simulink model "Demo_PreScanCarRealTime2D_cs"  (Simulink 8.0)
settings: Solver=ode5  SolverMode=SingleTasking  StartTime=0.0  StopTime=inf  FixedStep=1/1000  RelTol=1e-3
PreLoadFcn:
  | check_NUTS_defined;
  | assignin('base',['model_closed_' bdroot],false);
  | addpath('../includes_AEBS');
InitFcn:
  | PreScanExperimentInitFcn(bdroot)
StopFcn:
  | PreScanExperimentStopFcn(bdroot);
  | 
undefined symbols (not defined in the file or in its folder): DiscIntNeverNeededParam, Driver_Demands, vicrt_initcallback, vicrt_initmexcallback, vicrt_simulationmanager, x_ini_VRML, y_ini_VRML, z_ini_VRML
libraries: PreScanSimulinkLibrary x48, PreScanUsefulBlocks x15, simulink x15, simulink_extras x12, simulink_need_slupdate x1, dspsigops x1
(part 14 of 16)

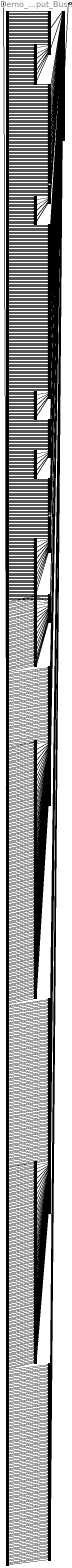
== system Demo_PreScanCarRealTime2D_cs/HostVehicle/Dynamics_UserSpecified/VI-CarRealTime/Output_Bus/IOmap2bus ==
  In : Inport
  Actual_Tread_Speed : BusCreator
  Aggregate_Force_At_Wheel : BusCreator
  Alpha : BusCreator
  Animator_Widget : BusCreator  Inputs=2
  Auxiliary_Roll_Force : BusCreator
  Auxiliary_Roll_Force_at_element : BusCreator  Inputs=2
  Auxiliary_Roll_deformation_at_element : BusCreator  Inputs=2
  Brake : BusCreator  Inputs=7
  Brake_Moment : BusCreator
  Caster_Angle : BusCreator  Inputs=2
  Chamber_Pressure : BusCreator
  Contact_Patch_Global_X : BusCreator
  Contact_Patch_Global_Y : BusCreator
  Contact_Patch_Global_Z : BusCreator
  Contact_Patch_Global_dZdX : BusCreator
  Contact_Patch_Global_dZdY : BusCreator
  Damper : BusCreator  Inputs=5
  Demux : Demux  Outputs=1108
  Drive_Moment : BusCreator
  Effective_Closing_Speed : BusCreator
  Effective_Penetration : BusCreator
  Effective_Rolling_Radius : BusCreator
  Equivalent_Tread_Speed : BusCreator
  Euler_Roll : BusCreator
  External_Friction_Scale_Factor : BusCreator
  Force_At_Bumper : BusCreator
  Force_At_Bumper1 : BusCreator
  Force_At_Damper : BusCreator
  Force_At_Spring : BusCreator
  Force_At_Wheel{,1..3} (4 alike) : BusCreator
  Force_Aux_At_Wheel : BusCreator
  Global_Omega_X : BusCreator
  Global_Omega_Y : BusCreator
  Global_Omega_Z : BusCreator
  Global_Vx : BusCreator
  Global_Vy : BusCreator
  Global_Vz : BusCreator
  Global_X : BusCreator
  Global_Y : BusCreator
  Global_Z : BusCreator
  Ground_Surface_Contact_Patch_Vx : BusCreator
  Ground_Surface_Contact_Patch_Vy : BusCreator
  Ground_Surface_Force_X : BusCreator
  Ground_Surface_Force_Y : BusCreator
  Ground_Surface_Force_Z : BusCreator
  Ground_Surface_Gyroscopic_Moment : BusCreator
  Ground_Surface_Kappa_Fy : BusCreator
  Ground_Surface_Moment_X : BusCreator
  Ground_Surface_Moment_Y : BusCreator
  Ground_Surface_Moment_Z : BusCreator
  Ground_Surface_Peak_Friction_X : BusCreator
  Ground_Surface_Peak_Friction_Y : BusCreator
  Ground_Surface_Residual_Torque : BusCreator
  Ground_Surface_Wheel_Center_Vx : BusCreator
  Gyro : BusCreator  Inputs=10
  Hook_rear : BusCreator  Inputs=18
  Hub_Carrier_Force_Global_X : BusCreator
  Hub_Carrier_Force_Global_Y : BusCreator
  Hub_Carrier_Force_Global_Z : BusCreator
  Hub_Carrier_Force_Local_X : BusCreator
  Hub_Carrier_Force_Local_Y : BusCreator
  Hub_Carrier_Force_Local_Z : BusCreator
  Hub_Carrier_Moment_Global_X : BusCreator
  Hub_Carrier_Moment_Global_Y : BusCreator
  Hub_Carrier_Moment_Global_Z : BusCreator
  Hub_Carrier_Moment_Local_X : BusCreator
  Hub_Carrier_Moment_Local_Y : BusCreator
  Hub_Carrier_Moment_Local_Z : BusCreator
  Hub_Carrier_Omega_X : BusCreator
  Hub_Carrier_Omega_Y : BusCreator
  Hub_Carrier_Omega_Z : BusCreator
  Hub_Carrier_Vx : BusCreator
  Hub_Carrier_Vy : BusCreator
  Hub_Carrier_Vz : BusCreator
  Inclination : BusCreator
  Inst_Stiff_Deflection : BusCreator
  Inst_Stiff_Force : BusCreator
  Jounce{,1..4} (5 alike) : BusCreator
  Jounce_Acceleration{,1..4} (5 alike) : BusCreator
  Jounce_Bumper : BusCreator  Inputs=5
  Jounce_Rate{,1..4} (5 alike) : BusCreator
  Kingpin_Moment : BusCreator  Inputs=1
  Kingpin_Moment1 : BusCreator  Inputs=2
  Lateral_Relaxation_Length : BusCreator
  Lateral_Slip_With_Lag : BusCreator
  Lateral_Slip_Without_Lag : BusCreator
  Loaded_Radius : BusCreator
  Locked_Damper_Moment : BusCreator
  Locked_Moment : BusCreator
  Locked_Spring_Moment : BusCreator
  Locked_Switch : BusCreator
  Lockup_Rotation : BusCreator
  Longitudinal_Relaxation_Length : BusCreator
  Longitudinal_Slip_With_Lag : BusCreator
  Longitudinal_Slip_Without_Lag : BusCreator
  Moment_Arm_Of_Fx : BusCreator
  Omega : BusCreator
  OutputBus : BusCreator  Inputs=92
  PID_CW_At_Spring : BusCreator
  PID_PH_At_Spring : BusCreator
  PID_RH_At_Spring : BusCreator
  Phi : BusCreator
  Pitch : BusCreator
  Pneumatic_Trail : BusCreator
  Psi : BusCreator
  Rebound_Bumper : BusCreator  Inputs=5
  Roll : BusCreator  Inputs=2
  Rotation : BusCreator
  Sensor : BusCreator  Inputs=16
  Side_View_Angle : BusCreator
  Spindle_Steer : BusCreator
  Spring : BusCreator  Inputs=10
  Steering_Instant_Axis_Rotation : BusCreator  Inputs=2
  Steering_System : BusCreator  Inputs=6
  Suspension : BusCreator  Inputs=14
  System : BusCreator
  System_Mass_Center : BusCreator  Inputs=13
  Theta : BusCreator
  TieRod_Force_Left : BusCreator  Inputs=3
  TieRod_Force_Right : BusCreator  Inputs=3
  Tire : BusCreator  Inputs=46
  User_Input_Contact_Patch_Global_Z_Perturbation : BusCreator
  Vehicle : BusCreator  Inputs=46
  Vehicle_States : BusCreator  Inputs=20
  Wheel : BusCreator  Inputs=37
  Yaw : BusCreator
  aero_forces : BusCreator  Inputs=24
  base_acceleration : BusCreator
  base_deformation : BusCreator
  base_velocity : BusCreator
  bul_jounce_stop_data : BusCreator  Inputs=6
  bul_rebound_stop_data : BusCreator  Inputs=6
  bur_jounce_stop_data : BusCreator  Inputs=6
  bur_rebound_stop_data : BusCreator  Inputs=6
  bus_dmp_jounce_stop_data : BusCreator  Inputs=6
  bus_ins_jounce_stop_data : BusCreator  Inputs=6
  bus_roll_dmp_jounce_stop_data : BusCreator  Inputs=6
  bus_spr_jounce_stop_data : BusCreator  Inputs=6
  chassis_accelerations : BusCreator  Inputs=6
  chassis_displacements : BusCreator  Inputs=9
  chassis_velocities : BusCreator  Inputs=6
  clutch : BusCreator  Inputs=2
  condition_sensors : BusCreator  Inputs=1
  cp_force_lateralFL : BusCreator  Inputs=3
  cp_force_lateralFR : BusCreator  Inputs=3
  cp_force_lateralRL : BusCreator  Inputs=3
  cp_force_lateralRR : BusCreator  Inputs=3
  cp_force_longitudinalFL : BusCreator  Inputs=3
  cp_force_longitudinalFR : BusCreator  Inputs=3
  cp_force_longitudinalRL : BusCreator  Inputs=3
  cp_force_longitudinalRR : BusCreator  Inputs=3
  cp_force_normalFL : BusCreator  Inputs=3
  cp_force_normalFR : BusCreator  Inputs=3
  cp_force_normalRL : BusCreator  Inputs=3
  cp_force_normalRR : BusCreator  Inputs=3
  cross_weight : BusCreator
  dal_ride_damper_data : BusCreator
  dar_ride_damper_data : BusCreator
  das_ride_damper_data : BusCreator  Inputs=6
  das_roll_damper_data : BusCreator  Inputs=8
  das_third_damper_data : BusCreator  Inputs=6
  differential_speeds : BusCreator  Inputs=6
  differential_torques : BusCreator  Inputs=6
  driver_demands : BusCreator  Inputs=7
  driving_machine_monitor : BusCreator  Inputs=9
  engine : BusCreator  Inputs=7
  fuel_consumption : BusCreator
  ges_chassis_XFORM : BusCreator  Inputs=6
  ges_hub_XFORMFL : BusCreator  Inputs=6
  ges_hub_XFORMFR : BusCreator  Inputs=6
  ges_hub_XFORMRL : BusCreator  Inputs=6
  ges_hub_XFORMRR : BusCreator  Inputs=6
  hub2cp_XFORMFL : BusCreator  Inputs=3
  hub2cp_XFORMFR : BusCreator  Inputs=3
  hub2cp_XFORMRL : BusCreator  Inputs=3
  hub2cp_XFORMRR : BusCreator  Inputs=3
  hub_center_accx : BusCreator
  hub_center_accy : BusCreator
  hub_center_accz : BusCreator
  ins_inerter_data : BusCreator  Inputs=10
  jfs_torsional_stiffness_data : BusCreator  Inputs=12
  nsl_ride_spring_data : BusCreator  Inputs=6
  nsr_ride_spring_data : BusCreator  Inputs=6
  nss_ride_spring_data : BusCreator  Inputs=10
  res_dmp_rebound_stop_data : BusCreator  Inputs=6
  res_ins_rebound_stop_data : BusCreator  Inputs=6
  res_roll_dmp_rebound_stop_data : BusCreator  Inputs=6
  res_spr_rebound_stop_data : BusCreator  Inputs=6
  rhl_front_setup_sensor_data : BusCreator  Inputs=1
  rhr_front_setup_sensor_data : BusCreator  Inputs=1
  rhs_rear_setup_sensor_data : BusCreator  Inputs=1
  til_wheel_tire_forces : BusCreator  Inputs=18
  til_wheel_tire_kinematics : BusCreator  Inputs=9
  til_wheel_tire_rolling_states : BusCreator  Inputs=9
  tir_wheel_tire_forces : BusCreator  Inputs=18
  tir_wheel_tire_kinematics : BusCreator  Inputs=9
  tir_wheel_tire_rolling_states : BusCreator  Inputs=9
  transmission : BusCreator
  ues_third_spring_data : BusCreator  Inputs=6
  upright_accelerometer : BusCreator
  wheel_angles : BusCreator  Inputs=12
  wheel_center_dispx : BusCreator
  wheel_center_dispy : BusCreator
  wheel_center_dispz : BusCreator
  whl_front_wheel_XFORM : BusCreator  Inputs=9
  whl_rear_wheel_XFORM : BusCreator  Inputs=9
  whl_twin_rear_wheel_XFORM : BusCreator  Inputs=9
  whr_front_wheel_XFORM : BusCreator  Inputs=9
  whr_rear_wheel_XFORM : BusCreator  Inputs=9
  whr_twin_rear_wheel_XFORM : BusCreator  Inputs=9
  Out : Outport
  wires:
  In/1 -> Demux/1
  OutputBus/1 -> Out/1  "OutputBus"
  aero_forces/1 -> OutputBus/1  "aero_forces"
  Demux/1 -> aero_forces/1  "aero_balance"
  Demux/2 -> aero_forces/2  "center_downforce"
  Demux/3 -> aero_forces/3  "center_sideforce"
  Demux/4 -> aero_forces/4  "drag_arm"
  Demux/5 -> aero_forces/5  "drag_force"
  Demux/6 -> aero_forces/6  "drag_force_front"
  Demux/7 -> aero_forces/7  "drag_force_rear"
  Demux/8 -> aero_forces/8  "drag_torque"
  Demux/9 -> aero_forces/9  "front_downforce"
  Demux/10 -> aero_forces/10  "front_sideforce"
  Demux/11 -> aero_forces/11  "height_auxiliary"
  Demux/12 -> aero_forces/12  "height_front"
  Demux/13 -> aero_forces/13  "height_rear"
  Demux/14 -> aero_forces/14  "lateral_velocity"
  Demux/15 -> aero_forces/15  "longitudinal_velocity"
  Demux/16 -> aero_forces/16  "pitch"
  Demux/17 -> aero_forces/17  "rear_downforce"
  Demux/18 -> aero_forces/18  "rear_sideforce"
  Demux/19 -> aero_forces/19  "roll"
  Demux/20 -> aero_forces/20  "roll_torque"
  Demux/21 -> aero_forces/21  "side_slip"
  Demux/22 -> aero_forces/22  "steer"
  Demux/23 -> aero_forces/23  "vertical_velocity"
  Demux/24 -> aero_forces/24  "yaw_torque"
  Brake/1 -> OutputBus/2  "Brake"
  Brake_Moment/1 -> Brake/1  "Brake_Moment"
  Demux/25 -> Brake_Moment/1
  Demux/26 -> Brake_Moment/2
  Demux/27 -> Brake_Moment/3  "R1"
  Demux/28 -> Brake_Moment/4  "R2"
  Chamber_Pressure/1 -> Brake/2  "Chamber_Pressure"
  Demux/29 -> Chamber_Pressure/1
  Demux/30 -> Chamber_Pressure/2
  Demux/31 -> Chamber_Pressure/3  "R1"
  Demux/32 -> Chamber_Pressure/4  "R2"
  Locked_Damper_Moment/1 -> Brake/3  "Locked_Damper_Moment"
  Demux/33 -> Locked_Damper_Moment/1
  Demux/34 -> Locked_Damper_Moment/2
  Demux/35 -> Locked_Damper_Moment/3  "R1"
  Demux/36 -> Locked_Damper_Moment/4  "R2"
  Locked_Moment/1 -> Brake/4  "Locked_Moment"
  Demux/37 -> Locked_Moment/1
  Demux/38 -> Locked_Moment/2
  Demux/39 -> Locked_Moment/3  "R1"
  Demux/40 -> Locked_Moment/4  "R2"
  Locked_Spring_Moment/1 -> Brake/5  "Locked_Spring_Moment"
  Demux/41 -> Locked_Spring_Moment/1
  Demux/42 -> Locked_Spring_Moment/2
  Demux/43 -> Locked_Spring_Moment/3  "R1"
  Demux/44 -> Locked_Spring_Moment/4  "R2"
  Locked_Switch/1 -> Brake/6  "Locked_Switch"
  Demux/45 -> Locked_Switch/1
  Demux/46 -> Locked_Switch/2
  Demux/47 -> Locked_Switch/3  "R1"
  Demux/48 -> Locked_Switch/4  "R2"
  Lockup_Rotation/1 -> Brake/7  "Lockup_Rotation"
  Demux/49 -> Lockup_Rotation/1
  Demux/50 -> Lockup_Rotation/2
  Demux/51 -> Lockup_Rotation/3  "R1"
  Demux/52 -> Lockup_Rotation/4  "R2"
  bul_jounce_stop_data/1 -> OutputBus/3  "bul_jounce_stop_data"
  Demux/53 -> bul_jounce_stop_data/1  "force_front"
  Demux/54 -> bul_jounce_stop_data/2  "force_rear"
  Demux/55 -> bul_jounce_stop_data/3  "jounce_front"
  Demux/56 -> bul_jounce_stop_data/4  "jounce_rear"
  Demux/57 -> bul_jounce_stop_data/5  "velocity_front"
  Demux/58 -> bul_jounce_stop_data/6  "velocity_rear"
  bul_rebound_stop_data/1 -> OutputBus/4  "bul_rebound_stop_data"
  Demux/59 -> bul_rebound_stop_data/1  "force_front"
  Demux/60 -> bul_rebound_stop_data/2  "force_rear"
  Demux/61 -> bul_rebound_stop_data/3  "jounce_front"
  Demux/62 -> bul_rebound_stop_data/4  "jounce_rear"
  Demux/63 -> bul_rebound_stop_data/5  "velocity_front"
  Demux/64 -> bul_rebound_stop_data/6  "velocity_rear"
  bur_jounce_stop_data/1 -> OutputBus/5  "bur_jounce_stop_data"
  Demux/65 -> bur_jounce_stop_data/1  "force_front"
  Demux/66 -> bur_jounce_stop_data/2  "force_rear"
  Demux/67 -> bur_jounce_stop_data/3  "jounce_front"
  Demux/68 -> bur_jounce_stop_data/4  "jounce_rear"
  Demux/69 -> bur_jounce_stop_data/5  "velocity_front"
  Demux/70 -> bur_jounce_stop_data/6  "velocity_rear"
  bur_rebound_stop_data/1 -> OutputBus/6  "bur_rebound_stop_data"
  Demux/71 -> bur_rebound_stop_data/1  "force_front"
  Demux/72 -> bur_rebound_stop_data/2  "force_rear"
  Demux/73 -> bur_rebound_stop_data/3  "jounce_front"
  Demux/74 -> bur_rebound_stop_data/4  "jounce_rear"
  Demux/75 -> bur_rebound_stop_data/5  "velocity_front"
  Demux/76 -> bur_rebound_stop_data/6  "velocity_rear"
  bus_dmp_jounce_stop_data/1 -> OutputBus/7  "bus_dmp_jounce_stop_data"
  Demux/77 -> bus_dmp_jounce_stop_data/1  "displacement_front"
  Demux/78 -> bus_dmp_jounce_stop_data/2  "displacement_rear"
  Demux/79 -> bus_dmp_jounce_stop_data/3  "force_front"
  Demux/80 -> bus_dmp_jounce_stop_data/4  "force_rear"
  Demux/81 -> bus_dmp_jounce_stop_data/5  "velocity_front"
  Demux/82 -> bus_dmp_jounce_stop_data/6  "velocity_rear"
  bus_ins_jounce_stop_data/1 -> OutputBus/8  "bus_ins_jounce_stop_data"
  Demux/83 -> bus_ins_jounce_stop_data/1  "displacement_front"
  Demux/84 -> bus_ins_jounce_stop_data/2  "displacement_rear"
  Demux/85 -> bus_ins_jounce_stop_data/3  "force_front"
  Demux/86 -> bus_ins_jounce_stop_data/4  "force_rear"
  Demux/87 -> bus_ins_jounce_stop_data/5  "velocity_front"
  Demux/88 -> bus_ins_jounce_stop_data/6  "velocity_rear"
  bus_roll_dmp_jounce_stop_data/1 -> OutputBus/9  "bus_roll_dmp_jounce_stop_data"
  Demux/89 -> bus_roll_dmp_jounce_stop_data/1  "displacement_front"
  Demux/90 -> bus_roll_dmp_jounce_stop_data/2  "displacement_rear"
  Demux/91 -> bus_roll_dmp_jounce_stop_data/3  "force_front"
  Demux/92 -> bus_roll_dmp_jounce_stop_data/4  "force_rear"
  Demux/93 -> bus_roll_dmp_jounce_stop_data/5  "velocity_front"
  Demux/94 -> bus_roll_dmp_jounce_stop_data/6  "velocity_rear"
  bus_spr_jounce_stop_data/1 -> OutputBus/10  "bus_spr_jounce_stop_data"
  Demux/95 -> bus_spr_jounce_stop_data/1  "displacement_front"
  Demux/96 -> bus_spr_jounce_stop_data/2  "displacement_rear"
  Demux/97 -> bus_spr_jounce_stop_data/3  "force_front"
  Demux/98 -> bus_spr_jounce_stop_data/4  "force_rear"
  Demux/99 -> bus_spr_jounce_stop_data/5  "velocity_front"
  Demux/100 -> bus_spr_jounce_stop_data/6  "velocity_rear"
  chassis_accelerations/1 -> OutputBus/11  "chassis_accelerations"
  Demux/101 -> chassis_accelerations/1  "lateral"
  Demux/102 -> chassis_accelerations/2  "longitudinal"
  Demux/103 -> chassis_accelerations/3  "pitch"
  Demux/104 -> chassis_accelerations/4  "roll"
  Demux/105 -> chassis_accelerations/5  "vertical"
  Demux/106 -> chassis_accelerations/6  "yaw"
  chassis_displacements/1 -> OutputBus/12  "chassis_displacements"
  Demux/107 -> chassis_displacements/1  "lateral"
  Demux/108 -> chassis_displacements/2  "longitudinal"
  Demux/109 -> chassis_displacements/3  "pitch"
  Demux/110 -> chassis_displacements/4  "pitch_wrt_road"
  Demux/111 -> chassis_displacements/5  "roll"
  Demux/112 -> chassis_displacements/6  "roll_wrt_road"
  Demux/113 -> chassis_displacements/7  "vertical"
  Demux/114 -> chassis_displacements/8  "vertical_wrt_road"
  Demux/115 -> chassis_displacements/9  "yaw"
  chassis_velocities/1 -> OutputBus/13  "chassis_velocities"
  Demux/116 -> chassis_velocities/1  "lateral"
  Demux/117 -> chassis_velocities/2  "longitudinal"
  Demux/118 -> chassis_velocities/3  "pitch"
  Demux/119 -> chassis_velocities/4  "roll"
  Demux/120 -> chassis_velocities/5  "vertical"
  Demux/121 -> chassis_velocities/6  "yaw"
  clutch/1 -> OutputBus/14  "clutch"
  Demux/122 -> clutch/1  "slip_angle"
  Demux/123 -> clutch/2  "torque"
  condition_sensors/1 -> OutputBus/15  "condition_sensors"
  Demux/124 -> condition_sensors/1  "side_slip_angle"
  cross_weight/1 -> OutputBus/16  "cross_weight"
  Demux/125 -> cross_weight/1  "crossL1R2_ratio"
  Demux/126 -> cross_weight/2  "crossR1L2_ratio"
  Demux/127 -> cross_weight/3  "nose_ratio"
  Demux/128 -> cross_weight/4  "side_right_ratio"
  dal_ride_damper_data/1 -> OutputBus/17  "dal_ride_damper_data"
  Demux/129 -> dal_ride_damper_data/1  "force_front"
  Demux/130 -> dal_ride_damper_data/2  "force_rear"
  Demux/131 -> dal_ride_damper_data/3  "velocity_front"
  Demux/132 -> dal_ride_damper_data/4  "velocity_rear"
  Damper/1 -> OutputBus/18  "Damper"
  Force_At_Damper/1 -> Damper/1  "Force_At_Damper"
  Demux/133 -> Force_At_Damper/1
  Demux/134 -> Force_At_Damper/2
  Demux/135 -> Force_At_Damper/3  "R1"
  Demux/136 -> Force_At_Damper/4  "R2"
  Force_At_Wheel/1 -> Damper/2  "Force_At_Wheel"
  Demux/137 -> Force_At_Wheel/1
  Demux/138 -> Force_At_Wheel/2
  Demux/139 -> Force_At_Wheel/3  "R1"
  Demux/140 -> Force_At_Wheel/4  "R2"
  Jounce/1 -> Damper/3  "Jounce"
  Demux/141 -> Jounce/1
  Demux/142 -> Jounce/2
  Demux/143 -> Jounce/3  "R1"
  Demux/144 -> Jounce/4  "R2"
  Jounce_Acceleration/1 -> Damper/4  "Jounce_Acceleration"
  Demux/145 -> Jounce_Acceleration/1
  Demux/146 -> Jounce_Acceleration/2
  Demux/147 -> Jounce_Acceleration/3  "R1"
  Demux/148 -> Jounce_Acceleration/4  "R2"
  Jounce_Rate/1 -> Damper/5  "Jounce_Rate"
  Demux/149 -> Jounce_Rate/1
  Demux/150 -> Jounce_Rate/2
  Demux/151 -> Jounce_Rate/3  "R1"
  Demux/152 -> Jounce_Rate/4  "R2"
  dar_ride_damper_data/1 -> OutputBus/19  "dar_ride_damper_data"
  Demux/153 -> dar_ride_damper_data/1  "force_front"
  Demux/154 -> dar_ride_damper_data/2  "force_rear"
  Demux/155 -> dar_ride_damper_data/3  "velocity_front"
  Demux/156 -> dar_ride_damper_data/4  "velocity_rear"
  das_ride_damper_data/1 -> OutputBus/20  "das_ride_damper_data"
  Demux/157 -> das_ride_damper_data/1  "displacement_front"
  Demux/158 -> das_ride_damper_data/2  "displacement_rear"
  Demux/159 -> das_ride_damper_data/3  "force_front"
  Demux/160 -> das_ride_damper_data/4  "force_rear"
  Demux/161 -> das_ride_damper_data/5  "velocity_front"
  Demux/162 -> das_ride_damper_data/6  "velocity_rear"
  das_roll_damper_data/1 -> OutputBus/21  "das_roll_damper_data"
  Demux/163 -> das_roll_damper_data/1  "displacement_front"
  Demux/164 -> das_roll_damper_data/2  "displacement_rear"
  Demux/165 -> das_roll_damper_data/3  "force_at_wheel_front"
  Demux/166 -> das_roll_damper_data/4  "force_at_wheel_rear"
  Demux/167 -> das_roll_damper_data/5  "force_front"
  Demux/168 -> das_roll_damper_data/6  "force_rear"
  Demux/169 -> das_roll_damper_data/7  "velocity_front"
  Demux/170 -> das_roll_damper_data/8  "velocity_rear"
  das_third_damper_data/1 -> OutputBus/22  "das_third_damper_data"
  Demux/171 -> das_third_damper_data/1  "displacement_front"
  Demux/172 -> das_third_damper_data/2  "displacement_rear"
  Demux/173 -> das_third_damper_data/3  "force_front"
  Demux/174 -> das_third_damper_data/4  "force_rear"
  Demux/175 -> das_third_damper_data/5  "velocity_front"
  Demux/176 -> das_third_damper_data/6  "velocity_rear"
  differential_speeds/1 -> OutputBus/23  "differential_speeds"
  Demux/177 -> differential_speeds/1  "halfshaft_omega_delta_front"
  Demux/178 -> differential_speeds/2  "halfshaft_omega_delta_rear"
  Demux/179 -> differential_speeds/3  "halfshaft_omega_left_front"
  Demux/180 -> differential_speeds/4  "halfshaft_omega_left_rear"
  Demux/181 -> differential_speeds/5  "halfshaft_omega_right_front"
  Demux/182 -> differential_speeds/6  "halfshaft_omega_right_rear"
  differential_torques/1 -> OutputBus/24  "differential_torques"
  Demux/183 -> differential_torques/1  "differential_torque_front"
  Demux/184 -> differential_torques/2  "differential_torque_rear"
  Demux/185 -> differential_torques/3  "output_torque_left_front"
  Demux/186 -> differential_torques/4  "output_torque_left_rear"
  Demux/187 -> differential_torques/5  "output_torque_right_front"
  Demux/188 -> differential_torques/6  "output_torque_right_rear"
  driver_demands/1 -> OutputBus/25  "driver_demands"
  Demux/189 -> driver_demands/1  "brake"
  Demux/190 -> driver_demands/2  "brake_pressure"
  Demux/191 -> driver_demands/3  "clutch"
  Demux/192 -> driver_demands/4  "gear"
  Demux/193 -> driver_demands/5  "steering"
  Demux/194 -> driver_demands/6  "steering_velocity"
  Demux/195 -> driver_demands/7  "throttle"
  driving_machine_monitor/1 -> OutputBus/26  "driving_machine_monitor"
  Demux/196 -> driving_machine_monitor/1  "maneuver_ID"
  Demux/197 -> driving_machine_monitor/2  "path_curvature"
  Demux/198 -> driving_machine_monitor/3  "path_distance"
  Demux/199 -> driving_machine_monitor/4  "path_s"
  Demux/200 -> driving_machine_monitor/5  "path_x"
  Demux/201 -> driving_machine_monitor/6  "path_y"
  Demux/202 -> driving_machine_monitor/7  "path_z"
  Demux/203 -> driving_machine_monitor/8  "target_ax"
  Demux/204 -> driving_machine_monitor/9  "target_vx"
  engine/1 -> OutputBus/27  "engine"
  Demux/205 -> engine/1  "effective_throttle"
  Demux/206 -> engine/2  "engine_rpm"
  Demux/207 -> engine/3  "Max_Engine_torque"
  Demux/208 -> engine/4  "Min_engine_torque"
  Demux/209 -> engine/5  "Power_HP"
  Demux/210 -> engine/6  "Power_KW"
  Demux/211 -> engine/7  "torque"
  fuel_consumption/1 -> OutputBus/28  "fuel_consumption"
  Demux/212 -> fuel_consumption/1  "BSFC"
  Demux/213 -> fuel_consumption/2  "instantaneous"
  Demux/214 -> fuel_consumption/3  "instantaneous_Liter_100km"
  Demux/215 -> fuel_consumption/4  "overall"
  ges_chassis_XFORM/1 -> OutputBus/29  "ges_chassis_XFORM"
  Demux/216 -> ges_chassis_XFORM/1  "Phi"
  Demux/217 -> ges_chassis_XFORM/2  "Psi"
  Demux/218 -> ges_chassis_XFORM/3  "Theta"
  Demux/219 -> ges_chassis_XFORM/4  "X"
  Demux/220 -> ges_chassis_XFORM/5  "Y"
  Demux/221 -> ges_chassis_XFORM/6  "Z"
  Gyro/1 -> OutputBus/30  "Gyro"
  Demux/222 -> Gyro/1  "Driver_X"
  Demux/223 -> Gyro/2  "Driver_Z"
  Demux/224 -> Gyro/3  "gyro_ACCX"
  Demux/225 -> Gyro/4  "gyro_ACCY"
  Demux/226 -> Gyro/5  "gyro_VX"
  Demux/227 -> Gyro/6  "gyro_X"
  Demux/228 -> Gyro/7  "gyro_Y"
  Demux/229 -> Gyro/8  "sensor_1_Z"
  Demux/230 -> Gyro/9  "sensor_2_Z"
  Demux/231 -> Gyro/10  "sensor_3_Z"
  Hook_rear/1 -> OutputBus/31  "Hook_rear"
  Demux/232 -> Hook_rear/1  "Global_force_X"
  Demux/233 -> Hook_rear/2  "Global_force_Y"
  Demux/234 -> Hook_rear/3  "Global_force_Z"
  Demux/235 -> Hook_rear/4  "Global_Moment_X"
  Demux/236 -> Hook_rear/5  "Global_Moment_Y"
  Demux/237 -> Hook_rear/6  "Global_Moment_Z"
  Demux/238 -> Hook_rear/7  "Hook_Phi"
  Demux/239 -> Hook_rear/8  "Hook_Psi"
  Demux/240 -> Hook_rear/9  "Hook_Theta"
  Demux/241 -> Hook_rear/10  "Hook_X"
  Demux/242 -> Hook_rear/11  "Hook_Y"
  Demux/243 -> Hook_rear/12  "Hook_Z"
  Demux/244 -> Hook_rear/13  "Local_force_X"
  Demux/245 -> Hook_rear/14  "Local_force_Y"
  Demux/246 -> Hook_rear/15  "Local_force_Z"
  Demux/247 -> Hook_rear/16  "Local_Moment_X"
  Demux/248 -> Hook_rear/17  "Local_Moment_Y"
  Demux/249 -> Hook_rear/18  "Local_Moment_Z"
  ins_inerter_data/1 -> OutputBus/32  "ins_inerter_data"
  Demux/250 -> ins_inerter_data/1  "acceleration_front"
  Demux/251 -> ins_inerter_data/2  "acceleration_rear"
  Demux/252 -> ins_inerter_data/3  "displacement_front"
  Demux/253 -> ins_inerter_data/4  "displacement_rear"
  Demux/254 -> ins_inerter_data/5  "force_at_wheel_front"
  Demux/255 -> ins_inerter_data/6  "force_at_wheel_rear"
  Demux/256 -> ins_inerter_data/7  "force_front"
  Demux/257 -> ins_inerter_data/8  "force_rear"
  Demux/258 -> ins_inerter_data/9  "velocity_front"
  Demux/259 -> ins_inerter_data/10  "velocity_rear"
  jfs_torsional_stiffness_data/1 -> OutputBus/33  "jfs_torsional_stiffness_data"
  Demux/260 -> jfs_torsional_stiffness_data/1  "AX_1"
  Demux/261 -> jfs_torsional_stiffness_data/2  "AY_1"
  Demux/262 -> jfs_torsional_stiffness_data/3  "AZ_1"
  Demux/263 -> jfs_torsional_stiffness_data/4  "FX_1"
  Demux/264 -> jfs_torsional_stiffness_data/5  "FY_1"
  Demux/265 -> jfs_torsional_stiffness_data/6  "FZ_1"
  Demux/266 -> jfs_torsional_stiffness_data/7  "TX_1"
  Demux/267 -> jfs_torsional_stiffness_data/8  "TY_1"
  Demux/268 -> jfs_torsional_stiffness_data/9  "TZ_1"
  Demux/269 -> jfs_torsional_stiffness_data/10  "X_1"
  Demux/270 -> jfs_torsional_stiffness_data/11  "Y_1"
  Demux/271 -> jfs_torsional_stiffness_data/12  "Z_1"
  Jounce_Bumper/1 -> OutputBus/34  "Jounce_Bumper"
  Force_At_Bumper/1 -> Jounce_Bumper/1  "Force_At_Bumper"
  Demux/272 -> Force_At_Bumper/1
  Demux/273 -> Force_At_Bumper/2
  Demux/274 -> Force_At_Bumper/3  "R1"
  Demux/275 -> Force_At_Bumper/4  "R2"
  Force_At_Wheel1/1 -> Jounce_Bumper/2  "Force_At_Wheel"
  Demux/276 -> Force_At_Wheel1/1
  Demux/277 -> Force_At_Wheel1/2
  Demux/278 -> Force_At_Wheel1/3  "R1"
  Demux/279 -> Force_At_Wheel1/4  "R2"
  Jounce1/1 -> Jounce_Bumper/3  "Jounce"
  Demux/280 -> Jounce1/1
  Demux/281 -> Jounce1/2
  Demux/282 -> Jounce1/3  "R1"
  Demux/283 -> Jounce1/4  "R2"
  Jounce_Acceleration1/1 -> Jounce_Bumper/4  "Jounce_Acceleration"
  Demux/284 -> Jounce_Acceleration1/1
  Demux/285 -> Jounce_Acceleration1/2
  Demux/286 -> Jounce_Acceleration1/3  "R1"
  Demux/287 -> Jounce_Acceleration1/4  "R2"
  Jounce_Rate1/1 -> Jounce_Bumper/5  "Jounce_Rate"
  Demux/288 -> Jounce_Rate1/1
  Demux/289 -> Jounce_Rate1/2
  Demux/290 -> Jounce_Rate1/3  "R1"
  Demux/291 -> Jounce_Rate1/4  "R2"
  nsl_ride_spring_data/1 -> OutputBus/35  "nsl_ride_spring_data"
  Demux/292 -> nsl_ride_spring_data/1  "displacement_front"
  Demux/293 -> nsl_ride_spring_data/2  "displacement_rear"
  Demux/294 -> nsl_ride_spring_data/3  "force_front"
  Demux/295 -> nsl_ride_spring_data/4  "force_rear"
  Demux/296 -> nsl_ride_spring_data/5  "velocity_front"
  Demux/297 -> nsl_ride_spring_data/6  "velocity_rear"
  nsr_ride_spring_data/1 -> OutputBus/36  "nsr_ride_spring_data"
  Demux/298 -> nsr_ride_spring_data/1  "displacement_front"
  Demux/299 -> nsr_ride_spring_data/2  "displacement_rear"
  Demux/300 -> nsr_ride_spring_data/3  "force_front"
  Demux/301 -> nsr_ride_spring_data/4  "force_rear"
  Demux/302 -> nsr_ride_spring_data/5  "velocity_front"
  Demux/303 -> nsr_ride_spring_data/6  "velocity_rear"
  nss_ride_spring_data/1 -> OutputBus/37  "nss_ride_spring_data"
  Demux/304 -> nss_ride_spring_data/1  "displacement_front"
  Demux/305 -> nss_ride_spring_data/2  "displacement_rear"
  Demux/306 -> nss_ride_spring_data/3  "force_at_wheel_front"
  Demux/307 -> nss_ride_spring_data/4  "force_at_wheel_rear"
  Demux/308 -> nss_ride_spring_data/5  "force_front"
  Demux/309 -> nss_ride_spring_data/6  "force_rear"
  Demux/310 -> nss_ride_spring_data/7  "PID_PH_At_Spring_front"
  Demux/311 -> nss_ride_spring_data/8  "PID_PH_At_Spring_rear"
  Demux/312 -> nss_ride_spring_data/9  "velocity_front"
  Demux/313 -> nss_ride_spring_data/10  "velocity_rear"
  Rebound_Bumper/1 -> OutputBus/38  "Rebound_Bumper"
  Force_At_Bumper1/1 -> Rebound_Bumper/1  "Force_At_Bumper"
  Demux/314 -> Force_At_Bumper1/1
  Demux/315 -> Force_At_Bumper1/2
  Demux/316 -> Force_At_Bumper1/3  "R1"
  Demux/317 -> Force_At_Bumper1/4  "R2"
  Force_At_Wheel2/1 -> Rebound_Bumper/2  "Force_At_Wheel"
  Demux/318 -> Force_At_Wheel2/1
  Demux/319 -> Force_At_Wheel2/2
  Demux/320 -> Force_At_Wheel2/3  "R1"
  Demux/321 -> Force_At_Wheel2/4  "R2"
  Jounce2/1 -> Rebound_Bumper/3  "Jounce"
  Demux/322 -> Jounce2/1
  Demux/323 -> Jounce2/2
  Demux/324 -> Jounce2/3  "R1"
  Demux/325 -> Jounce2/4  "R2"
  Jounce_Acceleration2/1 -> Rebound_Bumper/4  "Jounce_Acceleration"
  Demux/326 -> Jounce_Acceleration2/1
  Demux/327 -> Jounce_Acceleration2/2
  Demux/328 -> Jounce_Acceleration2/3  "R1"
  Demux/329 -> Jounce_Acceleration2/4  "R2"
  Jounce_Rate2/1 -> Rebound_Bumper/5  "Jounce_Rate"
  Demux/330 -> Jounce_Rate2/1
  Demux/331 -> Jounce_Rate2/2
  Demux/332 -> Jounce_Rate2/3  "R1"
  Demux/333 -> Jounce_Rate2/4  "R2"
  res_dmp_rebound_stop_data/1 -> OutputBus/39  "res_dmp_rebound_stop_data"
  Demux/334 -> res_dmp_rebound_stop_data/1  "displacement_front"
  Demux/335 -> res_dmp_rebound_stop_data/2  "displacement_rear"
  Demux/336 -> res_dmp_rebound_stop_data/3  "force_front"
  Demux/337 -> res_dmp_rebound_stop_data/4  "force_rear"
  Demux/338 -> res_dmp_rebound_stop_data/5  "velocity_front"
  Demux/339 -> res_dmp_rebound_stop_data/6  "velocity_rear"
  res_ins_rebound_stop_data/1 -> OutputBus/40  "res_ins_rebound_stop_data"
  Demux/340 -> res_ins_rebound_stop_data/1  "displacement_front"
  Demux/341 -> res_ins_rebound_stop_data/2  "displacement_rear"
  Demux/342 -> res_ins_rebound_stop_data/3  "force_front"
  Demux/343 -> res_ins_rebound_stop_data/4  "force_rear"
  Demux/344 -> res_ins_rebound_stop_data/5  "velocity_front"
  Demux/345 -> res_ins_rebound_stop_data/6  "velocity_rear"
  res_roll_dmp_rebound_stop_data/1 -> OutputBus/41  "res_roll_dmp_rebound_stop_data"
  Demux/346 -> res_roll_dmp_rebound_stop_data/1  "displacement_front"
  Demux/347 -> res_roll_dmp_rebound_stop_data/2  "displacement_rear"
  Demux/348 -> res_roll_dmp_rebound_stop_data/3  "force_front"
  Demux/349 -> res_roll_dmp_rebound_stop_data/4  "force_rear"
  Demux/350 -> res_roll_dmp_rebound_stop_data/5  "velocity_front"
  Demux/351 -> res_roll_dmp_rebound_stop_data/6  "velocity_rear"
  res_spr_rebound_stop_data/1 -> OutputBus/42  "res_spr_rebound_stop_data"
  Demux/352 -> res_spr_rebound_stop_data/1  "displacement_front"
  Demux/353 -> res_spr_rebound_stop_data/2  "displacement_rear"
  Demux/354 -> res_spr_rebound_stop_data/3  "force_front"
  Demux/355 -> res_spr_rebound_stop_data/4  "force_rear"
  Demux/356 -> res_spr_rebound_stop_data/5  "velocity_front"
  Demux/357 -> res_spr_rebound_stop_data/6  "velocity_rear"
  rhl_front_setup_sensor_data/1 -> OutputBus/43  "rhl_front_setup_sensor_data"
  Demux/358 -> rhl_front_setup_sensor_data/1  "ride_height"
  rhr_front_setup_sensor_data/1 -> OutputBus/44  "rhr_front_setup_sensor_data"
  Demux/359 -> rhr_front_setup_sensor_data/1  "ride_height"
  rhs_rear_setup_sensor_data/1 -> OutputBus/45  "rhs_rear_setup_sensor_data"
  Demux/360 -> rhs_rear_setup_sensor_data/1  "ride_height"
  Sensor/1 -> OutputBus/46  "Sensor"
  Demux/361 -> Sensor/1  "AccX_gyro"
  Demux/362 -> Sensor/2  "AccY_gyro"
  Demux/363 -> Sensor/3  "Ax"
  Demux/364 -> Sensor/4  "Ax_Without_Gravity"
  Demux/365 -> Sensor/5  "Ay"
  Demux/366 -> Sensor/6  "Ay_Without_Gravity"
  Demux/367 -> Sensor/7  "Az"
  Demux/368 -> Sensor/8  "Az_Without_Gravity"
  Demux/369 -> Sensor/9  "Global_X"
  Demux/370 -> Sensor/10  "Global_Y"
  Demux/371 -> Sensor/11  "Global_Z"
  Demux/372 -> Sensor/12  "Vx"
  Demux/373 -> Sensor/13  "Vx_gyro"
  Demux/374 -> Sensor/14  "Vy"
  Demux/375 -> Sensor/15  "Vz"
  Demux/376 -> Sensor/16  "YawRate_gyro"
  Spring/1 -> OutputBus/47  "Spring"
  Aggregate_Force_At_Wheel/1 -> Spring/1  "Aggregate_Force_At_Wheel"
  Demux/377 -> Aggregate_Force_At_Wheel/1
  Demux/378 -> Aggregate_Force_At_Wheel/2
  Demux/379 -> Aggregate_Force_At_Wheel/3  "R1"
  Demux/380 -> Aggregate_Force_At_Wheel/4  "R2"
  Force_At_Spring/1 -> Spring/2  "Force_At_Spring"
  Demux/381 -> Force_At_Spring/1
  Demux/382 -> Force_At_Spring/2
  Demux/383 -> Force_At_Spring/3  "R1"
  Demux/384 -> Force_At_Spring/4  "R2"
  Force_At_Wheel3/1 -> Spring/3  "Force_At_Wheel"
  Demux/385 -> Force_At_Wheel3/1
  Demux/386 -> Force_At_Wheel3/2
  Demux/387 -> Force_At_Wheel3/3  "R1"
  Demux/388 -> Force_At_Wheel3/4  "R2"
  Force_Aux_At_Wheel/1 -> Spring/4  "Force_Aux_At_Wheel"
  Demux/389 -> Force_Aux_At_Wheel/1
  Demux/390 -> Force_Aux_At_Wheel/2
  Demux/391 -> Force_Aux_At_Wheel/3  "R1"
  Demux/392 -> Force_Aux_At_Wheel/4  "R2"
  Jounce3/1 -> Spring/5  "Jounce"
  Demux/393 -> Jounce3/1
  Demux/394 -> Jounce3/2
  Demux/395 -> Jounce3/3  "R1"
  Demux/396 -> Jounce3/4  "R2"
  Jounce_Acceleration3/1 -> Spring/6  "Jounce_Acceleration"
  Demux/397 -> Jounce_Acceleration3/1
  Demux/398 -> Jounce_Acceleration3/2
  Demux/399 -> Jounce_Acceleration3/3  "R1"
  Demux/400 -> Jounce_Acceleration3/4  "R2"
  Jounce_Rate3/1 -> Spring/7  "Jounce_Rate"
  Demux/401 -> Jounce_Rate3/1
  Demux/402 -> Jounce_Rate3/2
  Demux/403 -> Jounce_Rate3/3  "R1"
  Demux/404 -> Jounce_Rate3/4  "R2"
  PID_CW_At_Spring/1 -> Spring/8  "PID_CW_At_Spring"
  Demux/405 -> PID_CW_At_Spring/1
  Demux/406 -> PID_CW_At_Spring/2
  Demux/407 -> PID_CW_At_Spring/3  "R1"
  Demux/408 -> PID_CW_At_Spring/4  "R2"
  PID_PH_At_Spring/1 -> Spring/9  "PID_PH_At_Spring"
  Demux/409 -> PID_PH_At_Spring/1
  Demux/410 -> PID_PH_At_Spring/2
  Demux/411 -> PID_PH_At_Spring/3  "R1"
  Demux/412 -> PID_PH_At_Spring/4  "R2"
  PID_RH_At_Spring/1 -> Spring/10  "PID_RH_At_Spring"
  Demux/413 -> PID_RH_At_Spring/1
  Demux/414 -> PID_RH_At_Spring/2
  Demux/415 -> PID_RH_At_Spring/3  "R1"
  Demux/416 -> PID_RH_At_Spring/4  "R2"
  Steering_System/1 -> OutputBus/48  "Steering_System"
  Demux/417 -> Steering_System/1  "Rack_displacement"
  Demux/418 -> Steering_System/2  "Rack_Force"
  Demux/419 -> Steering_System/3  "Steering_column_deformation"
  Demux/420 -> Steering_System/4  "Steering_Wheel_Torque"
  TieRod_Force_Left/1 -> Steering_System/5  "TieRod_Force_Left"
  Demux/421 -> TieRod_Force_Left/1  "X"
  Demux/422 -> TieRod_Force_Left/2  "Y"
  Demux/423 -> TieRod_Force_Left/3  "Z"
  TieRod_Force_Right/1 -> Steering_System/6  "TieRod_Force_Right"
  Demux/424 -> TieRod_Force_Right/1  "X"
  Demux/425 -> TieRod_Force_Right/2  "Y"
  Demux/426 -> TieRod_Force_Right/3  "Z"
  Suspension/1 -> OutputBus/49  "Suspension"
  Auxiliary_Roll_deformation_at_element/1 -> Suspension/1  "Auxiliary_Roll_deformation_at_element"
  Demux/427 -> Auxiliary_Roll_deformation_at_element/1  "SUSP1"
  Demux/428 -> Auxiliary_Roll_deformation_at_element/2  "SUSP2"
  Auxiliary_Roll_Force/1 -> Suspension/2  "Auxiliary_Roll_Force"
  Demux/429 -> Auxiliary_Roll_Force/1  "SUSP_L1"
  Demux/430 -> Auxiliary_Roll_Force/2  "SUSP_L2"
  Demux/431 -> Auxiliary_Roll_Force/3  "SUSP_R1"
  Demux/432 -> Auxiliary_Roll_Force/4  "SUSP_R2"
  Auxiliary_Roll_Force_at_element/1 -> Suspension/3  "Auxiliary_Roll_Force_at_element"
  Demux/433 -> Auxiliary_Roll_Force_at_element/1  "SUSP1"
  Demux/434 -> Auxiliary_Roll_Force_at_element/2  "SUSP2"
  base_acceleration/1 -> Suspension/4  "base_acceleration"
  Demux/435 -> base_acceleration/1
  Demux/436 -> base_acceleration/2
  Demux/437 -> base_acceleration/3  "R1"
  Demux/438 -> base_acceleration/4  "R2"
  base_deformation/1 -> Suspension/5  "base_deformation"
  Demux/439 -> base_deformation/1
  Demux/440 -> base_deformation/2
  Demux/441 -> base_deformation/3  "R1"
  Demux/442 -> base_deformation/4  "R2"
  base_velocity/1 -> Suspension/6  "base_velocity"
  Demux/443 -> base_velocity/1
  Demux/444 -> base_velocity/2
  Demux/445 -> base_velocity/3  "R1"
  Demux/446 -> base_velocity/4  "R2"
  Inst_Stiff_Deflection/1 -> Suspension/7  "Inst_Stiff_Deflection"
  Demux/447 -> Inst_Stiff_Deflection/1  "front_left"
  Demux/448 -> Inst_Stiff_Deflection/2  "front_right"
  Demux/449 -> Inst_Stiff_Deflection/3  "rear_left"
  Demux/450 -> Inst_Stiff_Deflection/4  "rear_right"
  Inst_Stiff_Force/1 -> Suspension/8  "Inst_Stiff_Force"
  Demux/451 -> Inst_Stiff_Force/1  "front_left"
  Demux/452 -> Inst_Stiff_Force/2  "front_right"
  Demux/453 -> Inst_Stiff_Force/3  "rear_left"
  Demux/454 -> Inst_Stiff_Force/4  "rear_right"
  Jounce4/1 -> Suspension/9  "Jounce"
  Demux/455 -> Jounce4/1
  Demux/456 -> Jounce4/2
  Demux/457 -> Jounce4/3  "R1"
  Demux/458 -> Jounce4/4  "R2"
  Jounce_Acceleration4/1 -> Suspension/10  "Jounce_Acceleration"
  Demux/459 -> Jounce_Acceleration4/1
  Demux/460 -> Jounce_Acceleration4/2
  Demux/461 -> Jounce_Acceleration4/3  "R1"
  Demux/462 -> Jounce_Acceleration4/4  "R2"
  Jounce_Rate4/1 -> Suspension/11  "Jounce_Rate"
  Demux/463 -> Jounce_Rate4/1
  Demux/464 -> Jounce_Rate4/2
  Demux/465 -> Jounce_Rate4/3  "R1"
  Demux/466 -> Jounce_Rate4/4  "R2"
  Kingpin_Moment/1 -> Suspension/12  "Kingpin_Moment"
  Demux/467 -> Kingpin_Moment/1  "SUSP1"
  Roll/1 -> Suspension/13  "Roll"
  Demux/468 -> Roll/1  "SUSP1"
  Demux/469 -> Roll/2  "SUSP2"
  Steering_Instant_Axis_Rotation/1 -> Suspension/14  "Steering_Instant_Axis_Rotation"
  Demux/470 -> Steering_Instant_Axis_Rotation/1